simulink model "ClimaManModMgr"  (R2022b)
settings: Solver=FixedStepDiscrete  StartTime=0.0  StopTime=10.0  FixedStep=0.2  RelTol=1e-3
(part 2 of 2)

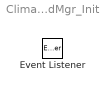
== system ClimaManModMgr/ClimaManModMgr_Init ==
  Event Listener : EventListener  EventName=reset

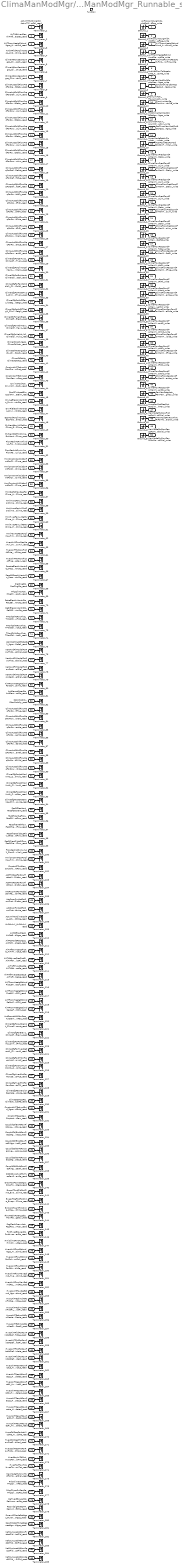
== system ClimaManModMgr/ClimaManModMgr_Runnable_sys ==
  ActvnTiForClimaCtrl_ActvnTiForClimaCtrl_read : Inport
  AirTAftHvacDes_AirTAftHvacDes_read : Inport  Port=2
  AirTFromHeatgEstimd_Ngcc_AirTFromHeatgEstimd_read : Inport  Port=3
  AmbTFildForClimaNgcc_AmbTFildForClima_read : Inport  Port=4
  ClimaAtRowSecActvNgcc_ClimaAtRowSecActv_read : Inport  Port=5
  ClimaAtRowSecActvNgcc_ClimaAtRowSecActvLe_read : Inport  Port=6
  ClimaAtRowSecActvNgcc_ClimaAtRowSecActvRi_read : Inport  Port=7
  ClimaAutCtrldFlowPredForDefrstAtStaby_ClimaAutCtrldFlowPredForDefrstAtStaby_read : Inport  Port=8
  ClimaAutCtrldFlowPredForDefrstNoLim_ClimaAutCtrldFlowPredForDefrstNoLim_read : Inport  Port=9
  ClimaAutCtrldFlowPredForRowFirstAtFlrAtStaby_ClimaAutCtrldFlowPredForRowFirstAtFlrAtStaby_read : Inport  Port=10
  ClimaAutCtrldFlowPredForRowFirstAtFlrNoLim_ClimaAutCtrldFlowPredForRowFirstAtFlrNoLim_read : Inport  Port=11
  ClimaAutCtrldFlowPredForRowFirstAtVentAtStaby_ClimaAutCtrldFlowPredForRowFirstAtVentAtStaby_read : Inport  Port=12
  ClimaAutCtrldFlowPredForRowFirstAtVentNoLim_ClimaAutCtrldFlowPredForRowFirstAtVentNoLim_read : Inport  Port=13
  ClimaAutCtrldFlowPredForRowSecAtFlrAtStaby_ClimaAutCtrldFlowPredForRowSecAtFlrAtStaby_read : Inport  Port=14
  ClimaAutCtrldFlowPredForRowSecAtFlrNoLim_ClimaAutCtrldFlowPredForRowSecAtFlrNoLim_read : Inport  Port=15
  ClimaAutCtrldFlowPredForRowSecAtVentAtStaby_ClimaAutCtrldFlowPredForRowSecAtVentAtStaby_read : Inport  Port=16
  ClimaAutCtrldFlowPredForRowSecAtVentNoLim_ClimaAutCtrldFlowPredForRowSecAtVentNoLim_read : Inport  Port=17
  ClimaAutCtrldFlowReqForDefrst_ClimaAutCtrldFlowReqForDefrst_read : Inport  Port=18
  ClimaAutCtrldFlowReqForDefrst_ClimaAutCtrldFlowReqForDefrstLe_read : Inport  Port=19
  ClimaAutCtrldFlowReqForDefrst_ClimaAutCtrldFlowReqForDefrstRi_read : Inport  Port=20
  ClimaAutCtrldFlowReqForRowFirstAtFlr_ClimaAutCtrldFlowReqForRowFirstAtFlr_read : Inport  Port=21
  ClimaAutCtrldFlowReqForRowFirstAtFlr_ClimaAutCtrldFlowReqForRowFirstAtFlrRi_read : Inport  Port=22
  ClimaAutCtrldFlowReqForRowSecAtFlr_ClimaAutCtrldFlowReqForRowSecAtFlr_read : Inport  Port=23
  ClimaAutCtrldFlowReqForRowSecAtFlr_ClimaAutCtrldFlowReqForRowSecAtFlrLe_read : Inport  Port=24
  ClimaAutCtrldFlowReqForRowSecAtFlr_ClimaAutCtrldFlowReqForRowSecAtFlrRi_read : Inport  Port=25
  ClimaAutCtrldFlowReqForRowSecAtVent_ClimaAutCtrldFlowReqForRowSecAtVent_read : Inport  Port=26
  ClimaAutCtrldFlowReqForRowSecAtVent_ClimaAutCtrldFlowReqForRowSecAtVentLe_read : Inport  Port=27
  ClimaAutCtrldFlowReqForRowSecAtVent_ClimaAutCtrldFlowReqForRowSecAtVentRi_read : Inport  Port=28
  ClimaCfgForCarTyp1_ClimaCfgForCarTyp1_read : Inport  Port=29
  ClimaCfgForClimaCtrlMan1_ClimaCfgForClimaCtrlMan1_read : Inport  Port=30
  ClimaCfgForExtVentnClimaCtrl_ClimaCfgForExtVentnClimaCtrl_read : Inport  Port=31
  ClimaCfgForFanPotiCtrld1_ClimaCfgForFanPotiCtrld1_read : Inport  Port=32
  ClimaCfgForHeatrTyp1_ClimaCfgForHeatrTyp1_read : Inport  Port=33
  ClimaCfgForNrOfFanLvlSetg1_ClimaCfgForNrOfFanLvlSetg1_read : Inport  Port=34
  ClimaCfgForNrOfTSetg1_ClimaCfgForNrOfTSetg1_read : Inport  Port=35
  ClimaCfgForVehElec1_ClimaCfgForVehElec1_read : Inport  Port=36
  ClimaCfgForVehHev1_ClimaCfgForVehHev1_read : Inport  Port=37
  ClimaCfgForVehHyb1_ClimaCfgForVehHyb1_read : Inport  Port=38
  ClimaCtrlActvNgcc_ClimaCtrlActv_read : Inport  Port=39
  ClimaCtrlForEngStbActv_ClimaCtrlForEngStbActv_read : Inport  Port=40
  ClimaStStrtUp_ClimaStStrtUp_read : Inport  Port=41
  CmptmtAirTEstimdAtRowFirst_CmptmtAirTEstimdAtRowFirst_read : Inport  Port=42
  CmptmtAirTEstimdAtRowSec_CmptmtAirTEstimdAtRowSec_read : Inport  Port=43
  CnvincModActv_CnvincModActv_read : Inport  Port=44
  CooltTInHeatElm_CooltTInHeatElm_read : Inport  Port=45
  DrvrLeElecAirVentnSts_DrvrLeElecAirVentnSts_read : Inport  Port=46
  DrvrRiElecAirVentnSts_DrvrRiElecAirVentnSts_read : Inport  Port=47
  EcoModForClimaActv_EcoModForClimaActv_read : Inport  Port=48
  ExtVentFanVolStsFrmClima_ExtVentFanVolStsFrmClima_read : Inport  Port=49
  ExtVentStsFrmClima_ExtVentStsFrmClima_read : Inport  Port=50
  FlowRednAtPwrAllwdLo_FlowRednAtPwrAllwdLo_read : Inport  Port=51
  FlowRednAtPwrLvlLo_FlowRednAtPwrLvlLo_read : Inport  Port=52
  HmiCmptmtAirDistbnFrntForClima_HmiCmptmtAirDistbnFrntForClima_read : Inport  Port=53
  HmiCmptmtAirDistbnFrntForClima_HmiCmptmtAirDistbnFrntRiForClima_read : Inport  Port=54
  HmiCmptmtAirDistbnFrntForClima_HmiCmptmtAirDistbnReForClima_read : Inport  Port=55
  HmiCmptmtAirDistbnFrntForClima_HmiCmptmtAirDistbnReRiForClima_read : Inport  Port=56
  HmiDefrstMaxReqForClima_HmiDefrstMaxReqForClima_read : Inport  Port=57
  HmiHvacFanLvlFrntForClima_HmiHvacFanLvlFrntForClima_read : Inport  Port=58
  HmiHvacFanLvlFrntForClima_HmiHvacFanLvlFrntRiForClima_read : Inport  Port=59
  HmiHvacFanLvlReForClima_HmiHvacFanLvlReForClima_read : Inport  Port=60
  HmiHvacFanLvlReForClima_HmiHvacFanLvlReRiForClima_read : Inport  Port=61
  HmiMaxACReqForClima_HmiMaxACReqForClima_read : Inport  Port=62
  HvacAirMFlowReqMaxAvl_HvacAirMFlowReqMaxAvl_read : Inport  Port=63
  HvacAirTForRowFirstAtFlrLe_HvacAirTForRowFirstAtFlrLe_HvacAirTForRowFirstAtFlrLe_read : Inport  Port=64
  HvacAirTForRowFirstAtFlrLeRaw_HvacAirTForRowFirstAtFlrLeRaw_read : Inport  Port=65
  PassLeElecAirVentnSts_PassLeElecAirVentnSts_read : Inport  Port=66
  PassRiElecAirVentnSts_PassRiElecAirVentnSts_read : Inport  Port=67
  PreClngSts_PreClngSts_read : Inport  Port=68
  PrkgClimaActv_PrkgClimaActv_read : Inport  Port=69
  ReLeElecAirVentnSts_ReLeElecAirVentnSts_read : Inport  Port=70
  ReRiElecAirVentnSts_ReRiElecAirVentnSts_read : Inport  Port=71
  TModCtrlAtRowFirst_TModCtrlAtRowFirst_read : Inport  Port=72
  TModCtrlAtRowFirst_TModCtrlAtRowFirstLe_read : Inport  Port=73
  TModCtrlAtRowFirst_TModCtrlAtRowFirstRi_read : Inport  Port=74
  VehModMngtGlbSafe1_Ngcc_VehModMngtGlbSafe1_read : Inport  Port=75
  VentAndFlrModelForRowFirstLeForModelDial_VentAndFlrModelForRowFirstLeForModelDial_read : Inport  Port=76
  VentAndFlrModelForRowFirstRiForModelDial_VentAndFlrModelForRowFirstRiForModelDial_read : Inport  Port=77
  VentAndFlrModelForRowSecLeForModelDial_VentAndFlrModelForRowSecLeForModelDial_read : Inport  Port=78
  VentAndFlrModelForRowSecRiForModelDial_VentAndFlrModelForRowSecRiForModelDial_read : Inport  Port=79
  AirTFromHeatgEstimdForCCM_AirTFromHeatgEstimd_read : Inport  Port=80
  AutDemstOperSts_AutDemstOperSts_read : Inport  Port=81
  CdsnProblty_CdsnProblty_read : Inport  Port=82
  ClimaAutCtrldFlowReqForRowFirstAtFlr_ClimaAutCtrldFlowReqForRowFirstAtFlrLe_read : Inport  Port=83
  ClimaAutCtrldFlowReqForRowFirstAtVent_ClimaAutCtrldFlowReqForRowFirstAtVent_read : Inport  Port=84
  ClimaAutCtrldFlowReqForRowFirstAtVent_ClimaAutCtrldFlowReqForRowFirstAtVentLe_read : Inport  Port=85
  ClimaAutCtrldFlowReqForRowFirstAtVent_ClimaAutCtrldFlowReqForRowFirstAtVentLeMid_read : Inport  Port=86
  ClimaAutCtrldFlowReqForRowFirstAtVent_ClimaAutCtrldFlowReqForRowFirstAtVentLeSide_read : Inport  Port=87
  ClimaAutCtrldFlowReqForRowFirstAtVent_ClimaAutCtrldFlowReqForRowFirstAtVentRi_read : Inport  Port=88
  ClimaAutCtrldFlowReqForRowFirstAtVent_ClimaAutCtrldFlowReqForRowFirstAtVentRiMid_read : Inport  Port=89
  ClimaAutCtrldFlowReqForRowFirstAtVent_ClimaAutCtrldFlowReqForRowFirstAtVentRiSide_read : Inport  Port=90
  ClimaCfgForActrModVrnt1_ClimaCfgForActrInd1_read : Inport  Port=91
  ClimaCfgForActrModVrnt1_ClimaCfgForActrModVrnt1_read : Inport  Port=92
  ClimaCfgForActrModVrnt1_ClimaCfgForActrModVrntCen_read : Inport  Port=93
  ClimaCfgForStatrtDevice_ClimaCfgForStartDevice_read : Inport  Port=94
  FacOfSecVent_FacOfSecVent_read : Inport  Port=95
  FacOfVentLeFlow_FacOfVentLeFlow_read : Inport  Port=96
  FacOfVentRiFlow_FacOfVentRiFlow_read : Inport  Port=97
  FacOfVentSideLeFlow_FacOfVentSideLeFlow_read : Inport  Port=98
  FacOfVentSideRiFlow_FacOfVentSideRiFlow_read : Inport  Port=99
  FlowRednAtPwrLvlLo1_FlowRednAtPwrLvlLo1_read : Inport  Port=100
  HmiCmptmtTSpForClima_HmiCmptmtTSpForClima_read : Inport  Port=101
  CmptmtTFrntRaw_CmptmtTFrntRaw_read : Inport  Port=102
  ActrFrntReqForRowFirstLeModDistbn_ActrFrntReqForRowFirstLeModDistbn_read : Inport  Port=103
  ActrFrntReqForRowFirstModDistbn_ActrFrntReqForRowFirstModDistbn_read : Inport  Port=104
  ActrPosnActForModDistbnRe_ActrPosnActForModDistbnRe_read : Inport  Port=105
  ActrPosnFrntActForRowFirstModDistbn_ActrPosnFrntActForRowFirstModDistbn_read : Inport  Port=106
  ActrPosnFrntActForRowFirstModDistbnLe_ActrPosnFrntActForRowFirstModDistbnLe_read : Inport  Port=107
  ActvnTiForClimaCtrlRe_ActvnTiForClimaCtrlRe_read : Inport  Port=108
  AirCdnAvl_AirCdnAvl_read : Inport  Port=109
  AirPtcEnadNgcc_AirPtcEnadNgcc_read : Inport  Port=110
  AirPtcModeReqNgcc_AirPtcModeReqNgcc_read : Inport  Port=111
  AirTAftHvacDesFirstLe_AirTAftHvacDesFirstLe_read : Inport  Port=112
  AirTAftHvacDesFirstRi_AirTAftHvacDesFirstRi_read : Inport  Port=113
  AirTAftHvacDesRe_AirTAftHvacDesRe_read : Inport  Port=114
  AirTAftHvacDesSecLe_AirTAftHvacDesSecLe_read : Inport  Port=115
  AirTFromHeatgEstimdFirstLeForCCM_AirTFromHeatgEstimdFirstLeForCCM_read : Inport  Port=116
  AirTFromHeatgEstimdFirstRiForCCM_AirTFromHeatgEstimdFirstRiForCCM_read : Inport  Port=117
  AirTFromHeatgEstimdReForCCM_AirTFromHeatgEstimdReForCCM_read : Inport  Port=118
  AirTFromHeatgEstimdSecLeForCCM_AirTFromHeatgEstimdSecLeForCCM_read : Inport  Port=119
  AutDemstWthFanReq_AutDemstWthFanReq_read : Inport  Port=120
  ClimaCfgForAutDemst_ClimaCfgForAutDemst_read : Inport  Port=121
  ClimaCfgForErev1_ClimaCfgForErev1_read : Inport  Port=122
  ClimaCfgForForHeatPmp_ClimaCfgForForHeatPmp_read : Inport  Port=123
  ClimaCfgForHvacRePrsnt1_ClimaCfgForHvacRePrsnt1_read : Inport  Port=124
  ClimaCfgForPTCInFrontHVAC_ClimaCfgForPTCInFrontHVAC_read : Inport  Port=125
  ClimaCfgForSnsrHumPrsnt1_ClimaCfgForSnsrHumPrsnt1_read : Inport  Port=126
  ClimaCfgHvacFrntForRowSecForActrTLe_ClimaCfgHvacFrntForRowSecForActrTLe_read : Inport  Port=127
  ClimaCfgHvacFrntForRowSecForActrTRi_ClimaCfgHvacFrntForRowSecForActrTRi_read : Inport  Port=128
  ClimaCfgReqVersionReplace_ClimaCfgReqVersionReplace_read : Inport  Port=129
  ClimaStStrtUpRe_ClimaStStrtUpRe_read : Inport  Port=130
  CmptmtAirTEstimdExtd_Ngcc_CmptmtAirTEstimdExtd_read : Inport  Port=131
  CmptmtTRearRaw_CmptmtTRearRaw_read : Inport  Port=132
  CoupldCtrlErrAtRowFirstAvg_CoupldCtrlErrAtRowFirstAvg_read : Inport  Port=133
  CoupldCtrlErrAtRowFirstLeNgcc_CoupldCtrlErrAtRowFirstLe_read : Inport  Port=134
  CoupldCtrlErrAtRowFirstRiNgcc_CoupldCtrlErrAtRowFirstRi_read : Inport  Port=135
  CoupldCtrlErrAtRowSecAvg_CoupldCtrlErrAtRowSecAvg_read : Inport  Port=136
  CoupldCtrlErrAtRowSecLeNgcc_CoupldCtrlErrAtRowSecLe_read : Inport  Port=137
  CoupldCtrlErrAtRowSecRiNgcc_CoupldCtrlErrAtRowSecRi_read : Inport  Port=138
  DCDCPwrAllwdForHvacFanRe_DCDCPwrAllwdForHvacFanRe_read : Inport  Port=139
  DropTopPosnStsNgcc_DropTopPosnStsNgcc_read : Inport  Port=140
  EvaprTFrntFildForClima_EvaprTFrntFildForClima_read : Inport  Port=141
  EvaprTReFildForClima_EvaprTReFildForClima_read : Inport  Port=142
  ExtVentToastFrmClima_Prkg_ExtVentToastFrmClima_read : Inport  Port=143
  FlowRednForEngStby_FlowRednForEngStby_read : Inport  Port=144
  FogFlashPrevnActv_FogFlashPrevnActv_read : Inport  Port=145
  FrntHvacBlowerSts_FrntHvacBlowerSts_read : Inport  Port=146
  HmiClimaFrntAutReq_HmiClimaFrntAutReq_read : Inport  Port=147
  HvacAirMFlowEstimd_Ngcc_HvacAirMFlowEstimd_read : Inport  Port=148
  HvacAirMFlowEstimdForCtrlr_HvacAirMFlowEstimdForCtrlr_read : Inport  Port=149
  HvacAirMFlowEstimdForCtrlrRe_HvacAirMFlowEstimdForCtrlrRe_read : Inport  Port=150
  HvacAirMFlowMaxEstimd_HvacAirMFlowMaxEstimd_read : Inport  Port=151
  HvacAirMFlowMaxEstimdRe_HvacAirMFlowMaxEstimdRe_read : Inport  Port=152
  HvacAirMFlowReEstimd_Ngcc_HvacAirMFlowReEstimd_read : Inport  Port=153
  HvacAirTEstimdAtRowFirstLe_HvacAirTEstimdAtRowFirstLe_read : Inport  Port=154
  HvacAirTEstimdAtRowFirstRi_HvacAirTEstimdAtRowFirstRi_read : Inport  Port=155
  HvacAirTEstimdAtRowSecLe_HvacAirTEstimdAtRowSecLe_read : Inport  Port=156
  HvacAirTEstimdAtRowSecRi_HvacAirTEstimdAtRowSecRi_read : Inport  Port=157
  HvacAirTFildForRowFirstAtDefrstLe_HvacAirTFildForRowFirstAtDefrstLe_read : Inport  Port=158
  HvacAirTFildForRowFirstAtDefrstRi_HvacAirTFildForRowFirstAtDefrstRi_read : Inport  Port=159
  HvacAirTFildForRowFirstAtDefrstSideLe_HvacAirTFildForRowFirstAtDefrstSideLe_read : Inport  Port=160
  HvacAirTFildForRowFirstAtDefrstSideRi_HvacAirTFildForRowFirstAtDefrstSideRi_read : Inport  Port=161
  HvacAirTReqAtRowFirstLe_HvacAirTReqAtRowFirstLe_read : Inport  Port=162
  HvacAirTReqAtRowFirstLe_HvacAirTReqAtRowFirstLe_IsUpdated_read : Inport  Port=163
  HvacAirTReqAtRowFirstRi_HvacAirTReqAtRowFirstRi_read : Inport  Port=164
  HvacAirTReqAtRowFirstRi_HvacAirTReqAtRowFirstRi_IsUpdated_read : Inport  Port=165
  HvacAirTReqAtRowSecLe_HvacAirTReqAtRowSecLe_read : Inport  Port=166
  HvacAirTReqAtRowSecLe_HvacAirTReqAtRowSecLe_IsUpdated_read : Inport  Port=167
  HvacAirTReqAtRowSecRi_HvacAirTReqAtRowSecRi_read : Inport  Port=168
  HvacAirTReqAtRowSecRi_HvacAirTReqAtRowSecRi_IsUpdated_read : Inport  Port=169
  HvacCtrlReqForActrModRe_HvacCtrlReqForActrModRe_read : Inport  Port=170
  HvacCtrlrReqFrntForRowFirstForActrMod_HvacCtrlrReqFrntForRowFirstForActrMod_read : Inport  Port=171
  HvacCtrlrReqFrntForRowFirstLeForActrMod_HvacCtrlrReqFrntForRowFirstLeForActrMod_read : Inport  Port=172
  HvacFanLvlFbFrnt_HvacFanLvlFbFrnt_read : Inport  Port=173
  HvacTarFlowTot_HvacTarFlowTot_read : Inport  Port=174
  ManModeForRowThrdForModelDial_ManModeForRowThrdForModelDial_read : Inport  Port=175
  PrkgClimaOnReq_PrkgClimaOnReq_read : Inport  Port=176
  PrkgClimaOnReqRe_PrkgClimaOnReqRe_read : Inport  Port=177
  ReHvacBlowerSts_ReHvacBlowerSts_read : Inport  Port=178
  ResrvdSigForECM4_ResrvdSigForECM4_read : Inport  Port=179
  RuranACModeSetNgcc_RuranACModeSetNgcc_read : Inport  Port=180
  SportModeThmgtRequestNgcc_SportModeThmgtRequestNgcc_read : Inport  Port=181
  VehSunLoadAtRowFirstLeFild_VehSunLoadAtRowFirstLeFild_read : Inport  Port=182
  VehSunLoadAtRowFirstRiFild_VehSunLoadAtRowFirstRiFild_read : Inport  Port=183
  VehSunLoadAtRowSecLeFild_VehSunLoadAtRowSecLeFild_read : Inport  Port=184
  VehSunLoadAtRowSecRiFild_VehSunLoadAtRowSecRiFild_read : Inport  Port=185
  function : TriggerPort  TriggerType=function-call  VariantControl=(inherit)  SampleTimeType=periodic  SampleTime=-1
  Ground_{1..7,9..50} (49 alike) : Ground
  Ground_8 : Subsystem
  Terminator_{1..185} (185 alike) : Terminator
  AirFlowLimDurgMidAmbtForNVHForHVACFrntFlag_AirFlowLimDurgMidAmbtForNVHForHVACFrntFlag_write : Outport
      settings: EnsureOutportIsVirtual=on
  AirFlowLimDurgMidAmbtForNVHForHVACReFlag_AirFlowLimDurgMidAmbtForNVHForHVACReFlag_write : Outport  Port=2
      settings: EnsureOutportIsVirtual=on
  AirTFromHeatgEstimdLimd_AirTFromHeatgEstimdLimd_write : Outport  Port=3
      settings: EnsureOutportIsVirtual=on
  AirTFromHeatgEstimdLimdRe_AirTFromHeatgEstimdLimdRe_write : Outport  Port=4
      settings: EnsureOutportIsVirtual=on
  AntiHotFlowToFaceActvFrnt_AntiHotFlowToFaceActvFrnt_write : Outport  Port=5
      settings: EnsureOutportIsVirtual=on
  AntiHotFlowToFaceActvRe_AntiHotFlowToFaceActvRe_write : Outport  Port=6
      settings: EnsureOutportIsVirtual=on
  ClimaManModCmftPopUpReqNgcc_ClimaManModCmftPopUpReqNgcc_write : Outport  Port=7
      settings: EnsureOutportIsVirtual=on
  CmptmtAirDistbnRspNgcc_CmptmtAirDistbnRspNgcc_write : Outport  Port=8
      settings: EnsureOutportIsVirtual=on
  FlowLimActv_FlowLimActv_write : Outport  Port=9
      settings: EnsureOutportIsVirtual=on
  FlowLimActvRe_FlowLimActvRe_write : Outport  Port=10
      settings: EnsureOutportIsVirtual=on
  FrntClimaManModCmftStsNgcc_FrntClimaManModCmftStsNgcc_write : Outport  Port=11
      settings: EnsureOutportIsVirtual=on
  LowrWtrTActvn_LowrWtrTActvn_write : Outport  Port=12
      settings: EnsureOutportIsVirtual=on
  ReClimaManModCmftStsNgcc_ReClimaManModCmftStsNgcc_write : Outport  Port=13
      settings: EnsureOutportIsVirtual=on
  RuranModeSetAirFlowActv_RuranModeSetAirFlowActv_write : Outport  Port=14
      settings: EnsureOutportIsVirtual=on
  ActrPosnReqForModDistbnForClimaCtrlMan_ActrPosnReqForModDistbnForClimaCtrlMan_write : Outport  Port=15
      settings: EnsureOutportIsVirtual=on
  ClimaFlowPredCorrdForManModForDefrstAtStaby_ClimaFlowPredCorrdForManModForDefrstAtStaby_write : Outport  Port=16
      settings: EnsureOutportIsVirtual=on
  ClimaFlowPredCorrdForManModForDefrstNoLim_ClimaFlowPredCorrdForManModForDefrstNoLim_write : Outport  Port=17
      settings: EnsureOutportIsVirtual=on
  ClimaFlowPredCorrdForManModForRowFirstAtFlrAtStaby_ClimaFlowPredCorrdForManModForRowFirstAtFlrAtStaby_write : Outport  Port=18
      settings: EnsureOutportIsVirtual=on
  ClimaFlowPredCorrdForManModForRowFirstAtFlrNoLim_ClimaFlowPredCorrdForManModForRowFirstAtFlrNoLim_write : Outport  Port=19
      settings: EnsureOutportIsVirtual=on
  ClimaFlowPredCorrdForManModForRowFirstAtVentAtStaby_ClimaFlowPredCorrdForManModForRowFirstAtVentAtStaby_write : Outport  Port=20
      settings: EnsureOutportIsVirtual=on
  ClimaFlowPredCorrdForManModForRowFirstAtVentNoLim_ClimaFlowPredCorrdForManModForRowFirstAtVentNoLim_write : Outport  Port=21
      settings: EnsureOutportIsVirtual=on
  ClimaFlowPredCorrdForManModForRowSecAtFlrAtStaby_ClimaFlowPredCorrdForManModForRowSecAtFlrAtStaby_write : Outport  Port=22
      settings: EnsureOutportIsVirtual=on
  ClimaFlowPredCorrdForManModForRowSecAtFlrNoLim_ClimaFlowPredCorrdForManModForRowSecAtFlrNoLim_write : Outport  Port=23
      settings: EnsureOutportIsVirtual=on
  ClimaFlowPredCorrdForManModForRowSecAtVentAtStaby_ClimaFlowPredCorrdForManModForRowSecAtVentAtStaby_write : Outport  Port=24
      settings: EnsureOutportIsVirtual=on
  ClimaFlowPredCorrdForManModForRowSecAtVentNoLim_ClimaFlowPredCorrdForManModForRowSecAtVentNoLim_write : Outport  Port=25
      settings: EnsureOutportIsVirtual=on
  ClimaFlowReqCorrdForManModForDefrst_ClimaFlowReqCorrdForManModForDefrst_write : Outport  Port=26
      settings: EnsureOutportIsVirtual=on
  ClimaFlowReqCorrdForManModForDefrst_ClimaFlowReqCorrdForManModForDefrstLe_write : Outport  Port=27
      settings: EnsureOutportIsVirtual=on
  ClimaFlowReqCorrdForManModForDefrst_ClimaFlowReqCorrdForManModForDefrstRi_write : Outport  Port=28
      settings: EnsureOutportIsVirtual=on
  ClimaFlowReqCorrdForManModForRowFirstAtFlr_ClimaFlowReqCorrdForManModForRowFirstAtFlr_write : Outport  Port=29
      settings: EnsureOutportIsVirtual=on
  ClimaFlowReqCorrdForManModForRowFirstAtFlr_ClimaFlowReqCorrdForManModForRowFirstAtFlrLe_write : Outport  Port=30
      settings: EnsureOutportIsVirtual=on
  ClimaFlowReqCorrdForManModForRowFirstAtFlr_ClimaFlowReqCorrdForManModForRowFirstAtFlrRi_write : Outport  Port=31
      settings: EnsureOutportIsVirtual=on
  ClimaFlowReqCorrdForManModForRowFirstAtVent_ClimaFlowReqCorrdForManModForRowFirstAtVent_write : Outport  Port=32
      settings: EnsureOutportIsVirtual=on
  ClimaFlowReqCorrdForManModForRowFirstAtVent_ClimaFlowReqCorrdForManModForRowFirstAtVentLe_write : Outport  Port=33
      settings: EnsureOutportIsVirtual=on
  ClimaFlowReqCorrdForManModForRowFirstAtVent_ClimaFlowReqCorrdForManModForRowFirstAtVentLeMid_write : Outport  Port=34
      settings: EnsureOutportIsVirtual=on
  ClimaFlowReqCorrdForManModForRowFirstAtVent_ClimaFlowReqCorrdForManModForRowFirstAtVentLeSide_write : Outport  Port=35
      settings: EnsureOutportIsVirtual=on
  ClimaFlowReqCorrdForManModForRowFirstAtVent_ClimaFlowReqCorrdForManModForRowFirstAtVentRi_write : Outport  Port=36
      settings: EnsureOutportIsVirtual=on
  ClimaFlowReqCorrdForManModForRowFirstAtVent_ClimaFlowReqCorrdForManModForRowFirstAtVentRiMid_write : Outport  Port=37
      settings: EnsureOutportIsVirtual=on
  ClimaFlowReqCorrdForManModForRowFirstAtVent_ClimaFlowReqCorrdForManModForRowFirstAtVentRiSide_write : Outport  Port=38
      settings: EnsureOutportIsVirtual=on
  ClimaFlowReqCorrdForManModForRowSecAtFlr_ClimaFlowReqCorrdForManModForRowSecAtFlr_write : Outport  Port=39
      settings: EnsureOutportIsVirtual=on
  ClimaFlowReqCorrdForManModForRowSecAtFlr_ClimaFlowReqCorrdForManModForRowSecAtFlrLe_write : Outport  Port=40
      settings: EnsureOutportIsVirtual=on
  ClimaFlowReqCorrdForManModForRowSecAtFlr_ClimaFlowReqCorrdForManModForRowSecAtFlrRi_write : Outport  Port=41
      settings: EnsureOutportIsVirtual=on
  ClimaFlowReqCorrdForManModForRowSecAtVent_ClimaFlowReqCorrdForManModForRowSecAtVent_write : Outport  Port=42
      settings: EnsureOutportIsVirtual=on
  ClimaFlowReqCorrdForManModForRowSecAtVent_ClimaFlowReqCorrdForManModForRowSecAtVentLe_write : Outport  Port=43
      settings: EnsureOutportIsVirtual=on
  ClimaFlowReqCorrdForManModForRowSecAtVent_ClimaFlowReqCorrdForManModForRowSecAtVentRi_write : Outport  Port=44
      settings: EnsureOutportIsVirtual=on
  FanManSetgActv_FanManSetgActv_write : Outport  Port=45
      settings: EnsureOutportIsVirtual=on
  HvacFanSpdReqFrntForClimaCtrlMan_HvacFanSpdReqFrntForClimaCtrlMan_write : Outport  Port=46
      settings: EnsureOutportIsVirtual=on
  ManModeForRowFirstLeForModelDial_ManModeForRowFirstLeForModelDial_write : Outport  Port=47
      settings: EnsureOutportIsVirtual=on
  ManModeForRowFirstRiForModelDial_ManModeForRowFirstRiForModelDial_write : Outport  Port=48
      settings: EnsureOutportIsVirtual=on
  ManModeForRowSecLeForModelDial_ManModeForRowSecLeForModelDial_write : Outport  Port=49
      settings: EnsureOutportIsVirtual=on
  ManModeForRowSecRiForModelDial_ManModeForRowSecRiForModelDial_write : Outport  Port=50
      settings: EnsureOutportIsVirtual=on
  wires:
  ActvnTiForClimaCtrl_ActvnTiForClimaCtrl_read/1 -> Terminator_1/1
  AirTAftHvacDes_AirTAftHvacDes_read/1 -> Terminator_2/1
  AirTFromHeatgEstimd_Ngcc_AirTFromHeatgEstimd_read/1 -> Terminator_3/1
  AmbTFildForClimaNgcc_AmbTFildForClima_read/1 -> Terminator_4/1
  ClimaAtRowSecActvNgcc_ClimaAtRowSecActv_read/1 -> Terminator_5/1
  ClimaAtRowSecActvNgcc_ClimaAtRowSecActvLe_read/1 -> Terminator_6/1
  ClimaAtRowSecActvNgcc_ClimaAtRowSecActvRi_read/1 -> Terminator_7/1
  ClimaAutCtrldFlowPredForDefrstAtStaby_ClimaAutCtrldFlowPredForDefrstAtStaby_read/1 -> Terminator_8/1
  ClimaAutCtrldFlowPredForDefrstNoLim_ClimaAutCtrldFlowPredForDefrstNoLim_read/1 -> Terminator_9/1
  ClimaAutCtrldFlowPredForRowFirstAtFlrAtStaby_ClimaAutCtrldFlowPredForRowFirstAtFlrAtStaby_read/1 -> Terminator_10/1
  ClimaAutCtrldFlowPredForRowFirstAtFlrNoLim_ClimaAutCtrldFlowPredForRowFirstAtFlrNoLim_read/1 -> Terminator_11/1
  ClimaAutCtrldFlowPredForRowFirstAtVentAtStaby_ClimaAutCtrldFlowPredForRowFirstAtVentAtStaby_read/1 -> Terminator_12/1
  ClimaAutCtrldFlowPredForRowFirstAtVentNoLim_ClimaAutCtrldFlowPredForRowFirstAtVentNoLim_read/1 -> Terminator_13/1
  ClimaAutCtrldFlowPredForRowSecAtFlrAtStaby_ClimaAutCtrldFlowPredForRowSecAtFlrAtStaby_read/1 -> Terminator_14/1
  ClimaAutCtrldFlowPredForRowSecAtFlrNoLim_ClimaAutCtrldFlowPredForRowSecAtFlrNoLim_read/1 -> Terminator_15/1
  ClimaAutCtrldFlowPredForRowSecAtVentAtStaby_ClimaAutCtrldFlowPredForRowSecAtVentAtStaby_read/1 -> Terminator_16/1
  ClimaAutCtrldFlowPredForRowSecAtVentNoLim_ClimaAutCtrldFlowPredForRowSecAtVentNoLim_read/1 -> Terminator_17/1
  ClimaAutCtrldFlowReqForDefrst_ClimaAutCtrldFlowReqForDefrst_read/1 -> Terminator_18/1
  ClimaAutCtrldFlowReqForDefrst_ClimaAutCtrldFlowReqForDefrstLe_read/1 -> Terminator_19/1
  ClimaAutCtrldFlowReqForDefrst_ClimaAutCtrldFlowReqForDefrstRi_read/1 -> Terminator_20/1
  ClimaAutCtrldFlowReqForRowFirstAtFlr_ClimaAutCtrldFlowReqForRowFirstAtFlr_read/1 -> Terminator_21/1
  ClimaAutCtrldFlowReqForRowFirstAtFlr_ClimaAutCtrldFlowReqForRowFirstAtFlrRi_read/1 -> Terminator_22/1
  ClimaAutCtrldFlowReqForRowSecAtFlr_ClimaAutCtrldFlowReqForRowSecAtFlr_read/1 -> Terminator_23/1
  ClimaAutCtrldFlowReqForRowSecAtFlr_ClimaAutCtrldFlowReqForRowSecAtFlrLe_read/1 -> Terminator_24/1
  ClimaAutCtrldFlowReqForRowSecAtFlr_ClimaAutCtrldFlowReqForRowSecAtFlrRi_read/1 -> Terminator_25/1
  ClimaAutCtrldFlowReqForRowSecAtVent_ClimaAutCtrldFlowReqForRowSecAtVent_read/1 -> Terminator_26/1
  ClimaAutCtrldFlowReqForRowSecAtVent_ClimaAutCtrldFlowReqForRowSecAtVentLe_read/1 -> Terminator_27/1
  ClimaAutCtrldFlowReqForRowSecAtVent_ClimaAutCtrldFlowReqForRowSecAtVentRi_read/1 -> Terminator_28/1
  ClimaCfgForCarTyp1_ClimaCfgForCarTyp1_read/1 -> Terminator_29/1
  ClimaCfgForClimaCtrlMan1_ClimaCfgForClimaCtrlMan1_read/1 -> Terminator_30/1
  ClimaCfgForExtVentnClimaCtrl_ClimaCfgForExtVentnClimaCtrl_read/1 -> Terminator_31/1
  ClimaCfgForFanPotiCtrld1_ClimaCfgForFanPotiCtrld1_read/1 -> Terminator_32/1
  ClimaCfgForHeatrTyp1_ClimaCfgForHeatrTyp1_read/1 -> Terminator_33/1
  ClimaCfgForNrOfFanLvlSetg1_ClimaCfgForNrOfFanLvlSetg1_read/1 -> Terminator_34/1
  ClimaCfgForNrOfTSetg1_ClimaCfgForNrOfTSetg1_read/1 -> Terminator_35/1
  ClimaCfgForVehElec1_ClimaCfgForVehElec1_read/1 -> Terminator_36/1
  ClimaCfgForVehHev1_ClimaCfgForVehHev1_read/1 -> Terminator_37/1
  ClimaCfgForVehHyb1_ClimaCfgForVehHyb1_read/1 -> Terminator_38/1
  ClimaCtrlActvNgcc_ClimaCtrlActv_read/1 -> Terminator_39/1
  ClimaCtrlForEngStbActv_ClimaCtrlForEngStbActv_read/1 -> Terminator_40/1
  ClimaStStrtUp_ClimaStStrtUp_read/1 -> Terminator_41/1
  CmptmtAirTEstimdAtRowFirst_CmptmtAirTEstimdAtRowFirst_read/1 -> Terminator_42/1
  CmptmtAirTEstimdAtRowSec_CmptmtAirTEstimdAtRowSec_read/1 -> Terminator_43/1
  CnvincModActv_CnvincModActv_read/1 -> Terminator_44/1
  CooltTInHeatElm_CooltTInHeatElm_read/1 -> Terminator_45/1
  DrvrLeElecAirVentnSts_DrvrLeElecAirVentnSts_read/1 -> Terminator_46/1
  DrvrRiElecAirVentnSts_DrvrRiElecAirVentnSts_read/1 -> Terminator_47/1
  EcoModForClimaActv_EcoModForClimaActv_read/1 -> Terminator_48/1
  ExtVentFanVolStsFrmClima_ExtVentFanVolStsFrmClima_read/1 -> Terminator_49/1
  ExtVentStsFrmClima_ExtVentStsFrmClima_read/1 -> Terminator_50/1
  FlowRednAtPwrAllwdLo_FlowRednAtPwrAllwdLo_read/1 -> Terminator_51/1
  FlowRednAtPwrLvlLo_FlowRednAtPwrLvlLo_read/1 -> Terminator_52/1
  HmiCmptmtAirDistbnFrntForClima_HmiCmptmtAirDistbnFrntForClima_read/1 -> Terminator_53/1
  HmiCmptmtAirDistbnFrntForClima_HmiCmptmtAirDistbnFrntRiForClima_read/1 -> Terminator_54/1
  HmiCmptmtAirDistbnFrntForClima_HmiCmptmtAirDistbnReForClima_read/1 -> Terminator_55/1
  HmiCmptmtAirDistbnFrntForClima_HmiCmptmtAirDistbnReRiForClima_read/1 -> Terminator_56/1
  HmiDefrstMaxReqForClima_HmiDefrstMaxReqForClima_read/1 -> Terminator_57/1
  HmiHvacFanLvlFrntForClima_HmiHvacFanLvlFrntForClima_read/1 -> Terminator_58/1
  HmiHvacFanLvlFrntForClima_HmiHvacFanLvlFrntRiForClima_read/1 -> Terminator_59/1
  HmiHvacFanLvlReForClima_HmiHvacFanLvlReForClima_read/1 -> Terminator_60/1
  HmiHvacFanLvlReForClima_HmiHvacFanLvlReRiForClima_read/1 -> Terminator_61/1
  HmiMaxACReqForClima_HmiMaxACReqForClima_read/1 -> Terminator_62/1
  HvacAirMFlowReqMaxAvl_HvacAirMFlowReqMaxAvl_read/1 -> Terminator_63/1
  HvacAirTForRowFirstAtFlrLe_HvacAirTForRowFirstAtFlrLe_HvacAirTForRowFirstAtFlrLe_read/1 -> Terminator_64/1
  HvacAirTForRowFirstAtFlrLeRaw_HvacAirTForRowFirstAtFlrLeRaw_read/1 -> Terminator_65/1
  PassLeElecAirVentnSts_PassLeElecAirVentnSts_read/1 -> Terminator_66/1
  PassRiElecAirVentnSts_PassRiElecAirVentnSts_read/1 -> Terminator_67/1
  PreClngSts_PreClngSts_read/1 -> Terminator_68/1
  PrkgClimaActv_PrkgClimaActv_read/1 -> Terminator_69/1
  ReLeElecAirVentnSts_ReLeElecAirVentnSts_read/1 -> Terminator_70/1
  ReRiElecAirVentnSts_ReRiElecAirVentnSts_read/1 -> Terminator_71/1
  TModCtrlAtRowFirst_TModCtrlAtRowFirst_read/1 -> Terminator_72/1
  TModCtrlAtRowFirst_TModCtrlAtRowFirstLe_read/1 -> Terminator_73/1
  TModCtrlAtRowFirst_TModCtrlAtRowFirstRi_read/1 -> Terminator_74/1
  VehModMngtGlbSafe1_Ngcc_VehModMngtGlbSafe1_read/1 -> Terminator_75/1
  VentAndFlrModelForRowFirstLeForModelDial_VentAndFlrModelForRowFirstLeForModelDial_read/1 -> Terminator_76/1
  VentAndFlrModelForRowFirstRiForModelDial_VentAndFlrModelForRowFirstRiForModelDial_read/1 -> Terminator_77/1
  VentAndFlrModelForRowSecLeForModelDial_VentAndFlrModelForRowSecLeForModelDial_read/1 -> Terminator_78/1
  VentAndFlrModelForRowSecRiForModelDial_VentAndFlrModelForRowSecRiForModelDial_read/1 -> Terminator_79/1
  AirTFromHeatgEstimdForCCM_AirTFromHeatgEstimd_read/1 -> Terminator_80/1
  AutDemstOperSts_AutDemstOperSts_read/1 -> Terminator_81/1
  CdsnProblty_CdsnProblty_read/1 -> Terminator_82/1
  ClimaAutCtrldFlowReqForRowFirstAtFlr_ClimaAutCtrldFlowReqForRowFirstAtFlrLe_read/1 -> Terminator_83/1
  ClimaAutCtrldFlowReqForRowFirstAtVent_ClimaAutCtrldFlowReqForRowFirstAtVent_read/1 -> Terminator_84/1
  ClimaAutCtrldFlowReqForRowFirstAtVent_ClimaAutCtrldFlowReqForRowFirstAtVentLe_read/1 -> Terminator_85/1
  ClimaAutCtrldFlowReqForRowFirstAtVent_ClimaAutCtrldFlowReqForRowFirstAtVentLeMid_read/1 -> Terminator_86/1
  ClimaAutCtrldFlowReqForRowFirstAtVent_ClimaAutCtrldFlowReqForRowFirstAtVentLeSide_read/1 -> Terminator_87/1
  ClimaAutCtrldFlowReqForRowFirstAtVent_ClimaAutCtrldFlowReqForRowFirstAtVentRi_read/1 -> Terminator_88/1
  ClimaAutCtrldFlowReqForRowFirstAtVent_ClimaAutCtrldFlowReqForRowFirstAtVentRiMid_read/1 -> Terminator_89/1
  ClimaAutCtrldFlowReqForRowFirstAtVent_ClimaAutCtrldFlowReqForRowFirstAtVentRiSide_read/1 -> Terminator_90/1
  ClimaCfgForActrModVrnt1_ClimaCfgForActrInd1_read/1 -> Terminator_91/1
  ClimaCfgForActrModVrnt1_ClimaCfgForActrModVrnt1_read/1 -> Terminator_92/1
  ClimaCfgForActrModVrnt1_ClimaCfgForActrModVrntCen_read/1 -> Terminator_93/1
  ClimaCfgForStatrtDevice_ClimaCfgForStartDevice_read/1 -> Terminator_94/1
  FacOfSecVent_FacOfSecVent_read/1 -> Terminator_95/1
  FacOfVentLeFlow_FacOfVentLeFlow_read/1 -> Terminator_96/1
  FacOfVentRiFlow_FacOfVentRiFlow_read/1 -> Terminator_97/1
  FacOfVentSideLeFlow_FacOfVentSideLeFlow_read/1 -> Terminator_98/1
  FacOfVentSideRiFlow_FacOfVentSideRiFlow_read/1 -> Terminator_99/1
  FlowRednAtPwrLvlLo1_FlowRednAtPwrLvlLo1_read/1 -> Terminator_100/1
  HmiCmptmtTSpForClima_HmiCmptmtTSpForClima_read/1 -> Terminator_101/1
  CmptmtTFrntRaw_CmptmtTFrntRaw_read/1 -> Terminator_102/1
  ActrFrntReqForRowFirstLeModDistbn_ActrFrntReqForRowFirstLeModDistbn_read/1 -> Terminator_103/1
  ActrFrntReqForRowFirstModDistbn_ActrFrntReqForRowFirstModDistbn_read/1 -> Terminator_104/1
  ActrPosnActForModDistbnRe_ActrPosnActForModDistbnRe_read/1 -> Terminator_105/1
  ActrPosnFrntActForRowFirstModDistbn_ActrPosnFrntActForRowFirstModDistbn_read/1 -> Terminator_106/1
  ActrPosnFrntActForRowFirstModDistbnLe_ActrPosnFrntActForRowFirstModDistbnLe_read/1 -> Terminator_107/1
  ActvnTiForClimaCtrlRe_ActvnTiForClimaCtrlRe_read/1 -> Terminator_108/1
  AirCdnAvl_AirCdnAvl_read/1 -> Terminator_109/1
  AirPtcEnadNgcc_AirPtcEnadNgcc_read/1 -> Terminator_110/1
  AirPtcModeReqNgcc_AirPtcModeReqNgcc_read/1 -> Terminator_111/1
  AirTAftHvacDesFirstLe_AirTAftHvacDesFirstLe_read/1 -> Terminator_112/1
  AirTAftHvacDesFirstRi_AirTAftHvacDesFirstRi_read/1 -> Terminator_113/1
  AirTAftHvacDesRe_AirTAftHvacDesRe_read/1 -> Terminator_114/1
  AirTAftHvacDesSecLe_AirTAftHvacDesSecLe_read/1 -> Terminator_115/1
  AirTFromHeatgEstimdFirstLeForCCM_AirTFromHeatgEstimdFirstLeForCCM_read/1 -> Terminator_116/1
  AirTFromHeatgEstimdFirstRiForCCM_AirTFromHeatgEstimdFirstRiForCCM_read/1 -> Terminator_117/1
  AirTFromHeatgEstimdReForCCM_AirTFromHeatgEstimdReForCCM_read/1 -> Terminator_118/1
  AirTFromHeatgEstimdSecLeForCCM_AirTFromHeatgEstimdSecLeForCCM_read/1 -> Terminator_119/1
  AutDemstWthFanReq_AutDemstWthFanReq_read/1 -> Terminator_120/1
  ClimaCfgForAutDemst_ClimaCfgForAutDemst_read/1 -> Terminator_121/1
  ClimaCfgForErev1_ClimaCfgForErev1_read/1 -> Terminator_122/1
  ClimaCfgForForHeatPmp_ClimaCfgForForHeatPmp_read/1 -> Terminator_123/1
  ClimaCfgForHvacRePrsnt1_ClimaCfgForHvacRePrsnt1_read/1 -> Terminator_124/1
  ClimaCfgForPTCInFrontHVAC_ClimaCfgForPTCInFrontHVAC_read/1 -> Terminator_125/1
  ClimaCfgForSnsrHumPrsnt1_ClimaCfgForSnsrHumPrsnt1_read/1 -> Terminator_126/1
  ClimaCfgHvacFrntForRowSecForActrTLe_ClimaCfgHvacFrntForRowSecForActrTLe_read/1 -> Terminator_127/1
  ClimaCfgHvacFrntForRowSecForActrTRi_ClimaCfgHvacFrntForRowSecForActrTRi_read/1 -> Terminator_128/1
  ClimaCfgReqVersionReplace_ClimaCfgReqVersionReplace_read/1 -> Terminator_129/1
  ClimaStStrtUpRe_ClimaStStrtUpRe_read/1 -> Terminator_130/1
  CmptmtAirTEstimdExtd_Ngcc_CmptmtAirTEstimdExtd_read/1 -> Terminator_131/1
  CmptmtTRearRaw_CmptmtTRearRaw_read/1 -> Terminator_132/1
  CoupldCtrlErrAtRowFirstAvg_CoupldCtrlErrAtRowFirstAvg_read/1 -> Terminator_133/1
  CoupldCtrlErrAtRowFirstLeNgcc_CoupldCtrlErrAtRowFirstLe_read/1 -> Terminator_134/1
  CoupldCtrlErrAtRowFirstRiNgcc_CoupldCtrlErrAtRowFirstRi_read/1 -> Terminator_135/1
  CoupldCtrlErrAtRowSecAvg_CoupldCtrlErrAtRowSecAvg_read/1 -> Terminator_136/1
  CoupldCtrlErrAtRowSecLeNgcc_CoupldCtrlErrAtRowSecLe_read/1 -> Terminator_137/1
  CoupldCtrlErrAtRowSecRiNgcc_CoupldCtrlErrAtRowSecRi_read/1 -> Terminator_138/1
  DCDCPwrAllwdForHvacFanRe_DCDCPwrAllwdForHvacFanRe_read/1 -> Terminator_139/1
  DropTopPosnStsNgcc_DropTopPosnStsNgcc_read/1 -> Terminator_140/1
  EvaprTFrntFildForClima_EvaprTFrntFildForClima_read/1 -> Terminator_141/1
  EvaprTReFildForClima_EvaprTReFildForClima_read/1 -> Terminator_142/1
  ExtVentToastFrmClima_Prkg_ExtVentToastFrmClima_read/1 -> Terminator_143/1
  FlowRednForEngStby_FlowRednForEngStby_read/1 -> Terminator_144/1
  FogFlashPrevnActv_FogFlashPrevnActv_read/1 -> Terminator_145/1
  FrntHvacBlowerSts_FrntHvacBlowerSts_read/1 -> Terminator_146/1
  HmiClimaFrntAutReq_HmiClimaFrntAutReq_read/1 -> Terminator_147/1
  HvacAirMFlowEstimd_Ngcc_HvacAirMFlowEstimd_read/1 -> Terminator_148/1
  HvacAirMFlowEstimdForCtrlr_HvacAirMFlowEstimdForCtrlr_read/1 -> Terminator_149/1
  HvacAirMFlowEstimdForCtrlrRe_HvacAirMFlowEstimdForCtrlrRe_read/1 -> Terminator_150/1
  HvacAirMFlowMaxEstimd_HvacAirMFlowMaxEstimd_read/1 -> Terminator_151/1
  HvacAirMFlowMaxEstimdRe_HvacAirMFlowMaxEstimdRe_read/1 -> Terminator_152/1
  HvacAirMFlowReEstimd_Ngcc_HvacAirMFlowReEstimd_read/1 -> Terminator_153/1
  HvacAirTEstimdAtRowFirstLe_HvacAirTEstimdAtRowFirstLe_read/1 -> Terminator_154/1
  HvacAirTEstimdAtRowFirstRi_HvacAirTEstimdAtRowFirstRi_read/1 -> Terminator_155/1
  HvacAirTEstimdAtRowSecLe_HvacAirTEstimdAtRowSecLe_read/1 -> Terminator_156/1
  HvacAirTEstimdAtRowSecRi_HvacAirTEstimdAtRowSecRi_read/1 -> Terminator_157/1
  HvacAirTFildForRowFirstAtDefrstLe_HvacAirTFildForRowFirstAtDefrstLe_read/1 -> Terminator_158/1
  HvacAirTFildForRowFirstAtDefrstRi_HvacAirTFildForRowFirstAtDefrstRi_read/1 -> Terminator_159/1
  HvacAirTFildForRowFirstAtDefrstSideLe_HvacAirTFildForRowFirstAtDefrstSideLe_read/1 -> Terminator_160/1
  HvacAirTFildForRowFirstAtDefrstSideRi_HvacAirTFildForRowFirstAtDefrstSideRi_read/1 -> Terminator_161/1
  HvacAirTReqAtRowFirstLe_HvacAirTReqAtRowFirstLe_read/1 -> Terminator_162/1
  HvacAirTReqAtRowFirstLe_HvacAirTReqAtRowFirstLe_IsUpdated_read/1 -> Terminator_163/1
  HvacAirTReqAtRowFirstRi_HvacAirTReqAtRowFirstRi_read/1 -> Terminator_164/1
  HvacAirTReqAtRowFirstRi_HvacAirTReqAtRowFirstRi_IsUpdated_read/1 -> Terminator_165/1
  HvacAirTReqAtRowSecLe_HvacAirTReqAtRowSecLe_read/1 -> Terminator_166/1
  HvacAirTReqAtRowSecLe_HvacAirTReqAtRowSecLe_IsUpdated_read/1 -> Terminator_167/1
  HvacAirTReqAtRowSecRi_HvacAirTReqAtRowSecRi_read/1 -> Terminator_168/1
  HvacAirTReqAtRowSecRi_HvacAirTReqAtRowSecRi_IsUpdated_read/1 -> Terminator_169/1
  HvacCtrlReqForActrModRe_HvacCtrlReqForActrModRe_read/1 -> Terminator_170/1
  HvacCtrlrReqFrntForRowFirstForActrMod_HvacCtrlrReqFrntForRowFirstForActrMod_read/1 -> Terminator_171/1
  HvacCtrlrReqFrntForRowFirstLeForActrMod_HvacCtrlrReqFrntForRowFirstLeForActrMod_read/1 -> Terminator_172/1
  HvacFanLvlFbFrnt_HvacFanLvlFbFrnt_read/1 -> Terminator_173/1
  HvacTarFlowTot_HvacTarFlowTot_read/1 -> Terminator_174/1
  ManModeForRowThrdForModelDial_ManModeForRowThrdForModelDial_read/1 -> Terminator_175/1
  PrkgClimaOnReq_PrkgClimaOnReq_read/1 -> Terminator_176/1
  PrkgClimaOnReqRe_PrkgClimaOnReqRe_read/1 -> Terminator_177/1
  ReHvacBlowerSts_ReHvacBlowerSts_read/1 -> Terminator_178/1
  ResrvdSigForECM4_ResrvdSigForECM4_read/1 -> Terminator_179/1
  RuranACModeSetNgcc_RuranACModeSetNgcc_read/1 -> Terminator_180/1
  SportModeThmgtRequestNgcc_SportModeThmgtRequestNgcc_read/1 -> Terminator_181/1
  VehSunLoadAtRowFirstLeFild_VehSunLoadAtRowFirstLeFild_read/1 -> Terminator_182/1
  VehSunLoadAtRowFirstRiFild_VehSunLoadAtRowFirstRiFild_read/1 -> Terminator_183/1
  VehSunLoadAtRowSecLeFild_VehSunLoadAtRowSecLeFild_read/1 -> Terminator_184/1
  VehSunLoadAtRowSecRiFild_VehSunLoadAtRowSecRiFild_read/1 -> Terminator_185/1
  Ground_1/1 -> AirFlowLimDurgMidAmbtForNVHForHVACFrntFlag_AirFlowLimDurgMidAmbtForNVHForHVACFrntFlag_write/1
  Ground_2/1 -> AirFlowLimDurgMidAmbtForNVHForHVACReFlag_AirFlowLimDurgMidAmbtForNVHForHVACReFlag_write/1
  Ground_3/1 -> AirTFromHeatgEstimdLimd_AirTFromHeatgEstimdLimd_write/1
  Ground_4/1 -> AirTFromHeatgEstimdLimdRe_AirTFromHeatgEstimdLimdRe_write/1
  Ground_5/1 -> AntiHotFlowToFaceActvFrnt_AntiHotFlowToFaceActvFrnt_write/1
  Ground_6/1 -> AntiHotFlowToFaceActvRe_AntiHotFlowToFaceActvRe_write/1
  Ground_7/1 -> ClimaManModCmftPopUpReqNgcc_ClimaManModCmftPopUpReqNgcc_write/1
  Ground_8/1(Out1) -> CmptmtAirDistbnRspNgcc_CmptmtAirDistbnRspNgcc_write/1
  Ground_9/1 -> FlowLimActv_FlowLimActv_write/1
  Ground_10/1 -> FlowLimActvRe_FlowLimActvRe_write/1
  Ground_11/1 -> FrntClimaManModCmftStsNgcc_FrntClimaManModCmftStsNgcc_write/1
  Ground_12/1 -> LowrWtrTActvn_LowrWtrTActvn_write/1
  Ground_13/1 -> ReClimaManModCmftStsNgcc_ReClimaManModCmftStsNgcc_write/1
  Ground_14/1 -> RuranModeSetAirFlowActv_RuranModeSetAirFlowActv_write/1
  Ground_15/1 -> ActrPosnReqForModDistbnForClimaCtrlMan_ActrPosnReqForModDistbnForClimaCtrlMan_write/1
  Ground_16/1 -> ClimaFlowPredCorrdForManModForDefrstAtStaby_ClimaFlowPredCorrdForManModForDefrstAtStaby_write/1
  Ground_17/1 -> ClimaFlowPredCorrdForManModForDefrstNoLim_ClimaFlowPredCorrdForManModForDefrstNoLim_write/1
  Ground_18/1 -> ClimaFlowPredCorrdForManModForRowFirstAtFlrAtStaby_ClimaFlowPredCorrdForManModForRowFirstAtFlrAtStaby_write/1
  Ground_19/1 -> ClimaFlowPredCorrdForManModForRowFirstAtFlrNoLim_ClimaFlowPredCorrdForManModForRowFirstAtFlrNoLim_write/1
  Ground_20/1 -> ClimaFlowPredCorrdForManModForRowFirstAtVentAtStaby_ClimaFlowPredCorrdForManModForRowFirstAtVentAtStaby_write/1
  Ground_21/1 -> ClimaFlowPredCorrdForManModForRowFirstAtVentNoLim_ClimaFlowPredCorrdForManModForRowFirstAtVentNoLim_write/1
  Ground_22/1 -> ClimaFlowPredCorrdForManModForRowSecAtFlrAtStaby_ClimaFlowPredCorrdForManModForRowSecAtFlrAtStaby_write/1
  Ground_23/1 -> ClimaFlowPredCorrdForManModForRowSecAtFlrNoLim_ClimaFlowPredCorrdForManModForRowSecAtFlrNoLim_write/1
  Ground_24/1 -> ClimaFlowPredCorrdForManModForRowSecAtVentAtStaby_ClimaFlowPredCorrdForManModForRowSecAtVentAtStaby_write/1
  Ground_25/1 -> ClimaFlowPredCorrdForManModForRowSecAtVentNoLim_ClimaFlowPredCorrdForManModForRowSecAtVentNoLim_write/1
  Ground_26/1 -> ClimaFlowReqCorrdForManModForDefrst_ClimaFlowReqCorrdForManModForDefrst_write/1
  Ground_27/1 -> ClimaFlowReqCorrdForManModForDefrst_ClimaFlowReqCorrdForManModForDefrstLe_write/1
  Ground_28/1 -> ClimaFlowReqCorrdForManModForDefrst_ClimaFlowReqCorrdForManModForDefrstRi_write/1
  Ground_29/1 -> ClimaFlowReqCorrdForManModForRowFirstAtFlr_ClimaFlowReqCorrdForManModForRowFirstAtFlr_write/1
  Ground_30/1 -> ClimaFlowReqCorrdForManModForRowFirstAtFlr_ClimaFlowReqCorrdForManModForRowFirstAtFlrLe_write/1
  Ground_31/1 -> ClimaFlowReqCorrdForManModForRowFirstAtFlr_ClimaFlowReqCorrdForManModForRowFirstAtFlrRi_write/1
  Ground_32/1 -> ClimaFlowReqCorrdForManModForRowFirstAtVent_ClimaFlowReqCorrdForManModForRowFirstAtVent_write/1
  Ground_33/1 -> ClimaFlowReqCorrdForManModForRowFirstAtVent_ClimaFlowReqCorrdForManModForRowFirstAtVentLe_write/1
  Ground_34/1 -> ClimaFlowReqCorrdForManModForRowFirstAtVent_ClimaFlowReqCorrdForManModForRowFirstAtVentLeMid_write/1
  Ground_35/1 -> ClimaFlowReqCorrdForManModForRowFirstAtVent_ClimaFlowReqCorrdForManModForRowFirstAtVentLeSide_write/1
  Ground_36/1 -> ClimaFlowReqCorrdForManModForRowFirstAtVent_ClimaFlowReqCorrdForManModForRowFirstAtVentRi_write/1
  Ground_37/1 -> ClimaFlowReqCorrdForManModForRowFirstAtVent_ClimaFlowReqCorrdForManModForRowFirstAtVentRiMid_write/1
  Ground_38/1 -> ClimaFlowReqCorrdForManModForRowFirstAtVent_ClimaFlowReqCorrdForManModForRowFirstAtVentRiSide_write/1
  Ground_39/1 -> ClimaFlowReqCorrdForManModForRowSecAtFlr_ClimaFlowReqCorrdForManModForRowSecAtFlr_write/1
  Ground_40/1 -> ClimaFlowReqCorrdForManModForRowSecAtFlr_ClimaFlowReqCorrdForManModForRowSecAtFlrLe_write/1
  Ground_41/1 -> ClimaFlowReqCorrdForManModForRowSecAtFlr_ClimaFlowReqCorrdForManModForRowSecAtFlrRi_write/1
  Ground_42/1 -> ClimaFlowReqCorrdForManModForRowSecAtVent_ClimaFlowReqCorrdForManModForRowSecAtVent_write/1
  Ground_43/1 -> ClimaFlowReqCorrdForManModForRowSecAtVent_ClimaFlowReqCorrdForManModForRowSecAtVentLe_write/1
  Ground_44/1 -> ClimaFlowReqCorrdForManModForRowSecAtVent_ClimaFlowReqCorrdForManModForRowSecAtVentRi_write/1
  Ground_45/1 -> FanManSetgActv_FanManSetgActv_write/1
  Ground_46/1 -> HvacFanSpdReqFrntForClimaCtrlMan_HvacFanSpdReqFrntForClimaCtrlMan_write/1
  Ground_47/1 -> ManModeForRowFirstLeForModelDial_ManModeForRowFirstLeForModelDial_write/1
  Ground_48/1 -> ManModeForRowFirstRiForModelDial_ManModeForRowFirstRiForModelDial_write/1
  Ground_49/1 -> ManModeForRowSecLeForModelDial_ManModeForRowSecLeForModelDial_write/1
  Ground_50/1 -> ManModeForRowSecRiForModelDial_ManModeForRowSecRiForModelDial_write/1

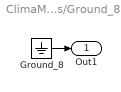
== system ClimaManModMgr/ClimaManModMgr_Runnable_sys/Ground_8 ==
  Ground_8 : Ground
  Out1 : Outport  OutDataTypeStr=Bus: CmptmtAirDistbnRsp  PortDimensions=1
  wires:
  Ground_8/1 -> Out1/1
